simulink library "Garbage"  (R2023a)
settings: Solver=ode45  StartTime=0.0  StopTime=10.0  RelTol=1e-3  EnableMultiTasking=on
undefined symbols (not defined in the file or in its folder): c, ending_column_index, ending_row_index, framebuffer_16x1, framebuffer_2x2, framebuffer_4x4, framebuffer_8x8, HEIGHT, hot, POS_X, POS_Y, starting_column_index, starting_row_index, WIDTH
libraries: Memory_Blocks_Lib x459, simulink x235, simulink_extras x132, dspmtrx3 x116, Bit_Multiplexers_Lib x67, Bit_Demultiplexers_Lib x49, Byte_Multiplexers_Lib x40, Miscellaneous x38, Computer_Library x17, Decoders_Lib x14, commutil2 x12, Arithmetic_Operations_Lib x8
(part 18 of 26)

== system Garbage/Stack_Pointer_Register ==
  Addr(2B) : Inport
  SetDirect(1b) : Inport  Port=2
  Decr(1b) : Inport  Port=3
  Incr(1b) : Inport  Port=4
  Clock : Inport  Port=5
  Bit to Integer Converter15 : simulink/Logic and Bit Operations/Bit to Integer Converter  nbits=16  bitOrder=MSB first  signedOutputValues=Unsigned  outDtype=uint16  outDtypeSigned=Inherit via internal rule
      settings: LibrarySourceBlock=|
      | commutil2/Bit to Integer
      | Converter
  Bit_0 : simulink_extras/Flip Flops/J-K Flip-Flop  initial_condition=bitand(c.STACK_START, bin2dec('0000 0000 0001'))
  Bit_1 : simulink_extras/Flip Flops/J-K Flip-Flop  initial_condition=bitand(c.STACK_START, bin2dec('0000 0000 0010'))
  Bit_10 : simulink_extras/Flip Flops/J-K Flip-Flop  initial_condition=bitand(c.STACK_START, bin2dec('0100 0000 0000'))
  Bit_11 : simulink_extras/Flip Flops/J-K Flip-Flop  initial_condition=bitand(c.STACK_START, bin2dec('1000 0000 0000'))
  Bit_2 : simulink_extras/Flip Flops/J-K Flip-Flop  initial_condition=bitand(c.STACK_START, bin2dec('0000 0000 0100'))
  Bit_3 : simulink_extras/Flip Flops/J-K Flip-Flop  initial_condition=bitand(c.STACK_START, bin2dec('0000 0000 1000'))
  Bit_4 : simulink_extras/Flip Flops/J-K Flip-Flop  initial_condition=bitand(c.STACK_START, bin2dec('0000 0001 0000'))
  Bit_5 : simulink_extras/Flip Flops/J-K Flip-Flop  initial_condition=bitand(c.STACK_START, bin2dec('0000 0010 0000'))
  Bit_6 : simulink_extras/Flip Flops/J-K Flip-Flop  initial_condition=bitand(c.STACK_START, bin2dec('0000 0100 0000'))
  Bit_7 : simulink_extras/Flip Flops/J-K Flip-Flop  initial_condition=bitand(c.STACK_START, bin2dec('0000 1000 0000'))
  Bit_8 : simulink_extras/Flip Flops/J-K Flip-Flop  initial_condition=bitand(c.STACK_START, bin2dec('0001 0000 0000'))
  Bit_9 : simulink_extras/Flip Flops/J-K Flip-Flop  initial_condition=bitand(c.STACK_START, bin2dec('0010 0000 0000'))
  Bit_Multiplexer_2_to_1(00) : Bit_Multiplexers_Lib/Bit_Multiplexers_Lib/Bit_Multiplexer_2_to_1
  Bit_Multiplexer_2_to_1(01) : Bit_Multiplexers_Lib/Bit_Multiplexers_Lib/Bit_Multiplexer_2_to_1
  Bit_Multiplexer_2_to_1(02) : Bit_Multiplexers_Lib/Bit_Multiplexers_Lib/Bit_Multiplexer_2_to_1
  Bit_Multiplexer_2_to_1(03) : Bit_Multiplexers_Lib/Bit_Multiplexers_Lib/Bit_Multiplexer_2_to_1
  Bit_Multiplexer_2_to_1(04) : Bit_Multiplexers_Lib/Bit_Multiplexers_Lib/Bit_Multiplexer_2_to_1
  Bit_Multiplexer_2_to_1(05) : Bit_Multiplexers_Lib/Bit_Multiplexers_Lib/Bit_Multiplexer_2_to_1
  Bit_Multiplexer_2_to_1(06) : Bit_Multiplexers_Lib/Bit_Multiplexers_Lib/Bit_Multiplexer_2_to_1
  Bit_Multiplexer_2_to_1(07) : Bit_Multiplexers_Lib/Bit_Multiplexers_Lib/Bit_Multiplexer_2_to_1
  Bit_Multiplexer_2_to_1(08) : Bit_Multiplexers_Lib/Bit_Multiplexers_Lib/Bit_Multiplexer_2_to_1
  Bit_Multiplexer_2_to_1(09) : Bit_Multiplexers_Lib/Bit_Multiplexers_Lib/Bit_Multiplexer_2_to_1
  Bit_Multiplexer_2_to_1(10) : Bit_Multiplexers_Lib/Bit_Multiplexers_Lib/Bit_Multiplexer_2_to_1
  Bit_Multiplexer_2_to_1(11) : Bit_Multiplexers_Lib/Bit_Multiplexers_Lib/Bit_Multiplexer_2_to_1
  Bit_Multiplexer_2_to_1(12) : Bit_Multiplexers_Lib/Bit_Multiplexers_Lib/Bit_Multiplexer_2_to_1
  Bit_Multiplexer_2_to_1(13) : Bit_Multiplexers_Lib/Bit_Multiplexers_Lib/Bit_Multiplexer_2_to_1
  Bit_Multiplexer_2_to_1(14) : Bit_Multiplexers_Lib/Bit_Multiplexers_Lib/Bit_Multiplexer_2_to_1
  Bit_Multiplexer_2_to_1(15) : Bit_Multiplexers_Lib/Bit_Multiplexers_Lib/Bit_Multiplexer_2_to_1
  Bit_Multiplexer_2_to_1(16) : Bit_Multiplexers_Lib/Bit_Multiplexers_Lib/Bit_Multiplexer_2_to_1
  Bit_Multiplexer_2_to_1(17) : Bit_Multiplexers_Lib/Bit_Multiplexers_Lib/Bit_Multiplexer_2_to_1
  Bit_Multiplexer_2_to_1(18) : Bit_Multiplexers_Lib/Bit_Multiplexers_Lib/Bit_Multiplexer_2_to_1
  Bit_Multiplexer_2_to_1(19) : Bit_Multiplexers_Lib/Bit_Multiplexers_Lib/Bit_Multiplexer_2_to_1
  Bit_Multiplexer_2_to_1(20) : Bit_Multiplexers_Lib/Bit_Multiplexers_Lib/Bit_Multiplexer_2_to_1
  Bit_Multiplexer_2_to_1(21) : Bit_Multiplexers_Lib/Bit_Multiplexers_Lib/Bit_Multiplexer_2_to_1
  Bit_Multiplexer_2_to_1(22) : Bit_Multiplexers_Lib/Bit_Multiplexers_Lib/Bit_Multiplexer_2_to_1
  Bit_Multiplexer_2_to_1(23) : Bit_Multiplexers_Lib/Bit_Multiplexers_Lib/Bit_Multiplexer_2_to_1
  Bit_Multiplexer_2_to_1(24) : Bit_Multiplexers_Lib/Bit_Multiplexers_Lib/Bit_Multiplexer_2_to_1
  Bit_Multiplexer_2_to_1(25) : Bit_Multiplexers_Lib/Bit_Multiplexers_Lib/Bit_Multiplexer_2_to_1
  Bit_Multiplexer_2_to_1(26) : Bit_Multiplexers_Lib/Bit_Multiplexers_Lib/Bit_Multiplexer_2_to_1
  Bit_Multiplexer_2_to_1(27) : Bit_Multiplexers_Lib/Bit_Multiplexers_Lib/Bit_Multiplexer_2_to_1
  Bit_Multiplexer_2_to_1(28) : Bit_Multiplexers_Lib/Bit_Multiplexers_Lib/Bit_Multiplexer_2_to_1
  Bit_Multiplexer_2_to_1(29) : Bit_Multiplexers_Lib/Bit_Multiplexers_Lib/Bit_Multiplexer_2_to_1
  Bit_Multiplexer_2_to_1(30) : Bit_Multiplexers_Lib/Bit_Multiplexers_Lib/Bit_Multiplexer_2_to_1
  Bit_Multiplexer_2_to_1(31) : Bit_Multiplexers_Lib/Bit_Multiplexers_Lib/Bit_Multiplexer_2_to_1
  Bit_Multiplexer_2_to_1(32) : Bit_Multiplexers_Lib/Bit_Multiplexers_Lib/Bit_Multiplexer_2_to_1
  Bit_Multiplexer_2_to_1(33) : Bit_Multiplexers_Lib/Bit_Multiplexers_Lib/Bit_Multiplexer_2_to_1
  Bit_Multiplexer_2_to_1(34) : Bit_Multiplexers_Lib/Bit_Multiplexers_Lib/Bit_Multiplexer_2_to_1
  Bus Creator : BusCreator  Inputs=16
  Bus Selector : BusSelector  OutputSignals=signal1,signal2,signal3,signal4,signal5,signal6,signal7,signal8,signal9,signal10,signal11,signal12,signal13,signal14,signal15,signal16
  Constant10 : Constant  Value=0  OutDataTypeStr=boolean
  Increment Enabled1 : Logic  IconShape=distinctive  AllPortsSameDT=off  OutDataTypeStr=boolean
  Increment Enabled2 : Logic  IconShape=distinctive  AllPortsSameDT=off  OutDataTypeStr=boolean
  Increment Enabled3 : Logic  IconShape=distinctive  AllPortsSameDT=off  OutDataTypeStr=boolean
  Logical Operator{1,5,9,14,18,25,27..28,30,33..34} (11 alike) : Logic  IconShape=distinctive  AllPortsSameDT=off  OutDataTypeStr=boolean
  Logical Operator{2..4,6..8,10..11,15..17,19..24,26,29,31..32,35..39} (26 alike) : Logic  Operator=NOT  IconShape=distinctive  AllPortsSameDT=off  OutDataTypeStr=boolean
  Terminator{1..16} (16 alike) : Terminator
  or : Logic  Operator=OR  IconShape=distinctive  AllPortsSameDT=off  OutDataTypeStr=boolean
  AddrOut(2B) : Outport
  tag scope: STACK_POINTER=global
  wires:
  Logical Operator18/1 -> Increment Enabled3/1, Bit_Multiplexer_2_to_1(13)/3, Bit_Multiplexer_2_to_1(12)/3
  Bus Selector/12 -> Bit_Multiplexer_2_to_1(12)/2, Logical Operator17/1  "<signal12>"
  Logical Operator17/1 -> Bit_Multiplexer_2_to_1(13)/2
  Bit_4/1 -> Bus Creator/12, Bit_Multiplexer_2_to_1(14)/2, Logical Operator23/1
  Bus Selector/9 -> Bit_Multiplexer_2_to_1(21)/2, Logical Operator37/1  "<signal9>"
  Logical Operator37/1 -> Bit_Multiplexer_2_to_1(22)/2
  Bit_Multiplexer_2_to_1(21)/1 -> Bit_7/1
  Bit_Multiplexer_2_to_1(22)/1 -> Bit_7/3
  Logical Operator16/1 -> Bit_Multiplexer_2_to_1(19)/2
  Bus Selector/10 -> Bit_Multiplexer_2_to_1(18)/2, Logical Operator16/1  "<signal10>"
  Bit_Multiplexer_2_to_1(19)/1 -> Bit_6/3
  Bit_Multiplexer_2_to_1(18)/1 -> Bit_6/1
  Bus Selector/11 -> Bit_Multiplexer_2_to_1(15)/2, Logical Operator15/1  "<signal11>"
  Logical Operator15/1 -> Bit_Multiplexer_2_to_1(16)/2
  Bit_Multiplexer_2_to_1(16)/1 -> Bit_5/3
  Bit_Multiplexer_2_to_1(15)/1 -> Bit_5/1
  Bit_Multiplexer_2_to_1(12)/1 -> Bit_4/1
  Bit_Multiplexer_2_to_1(13)/1 -> Bit_4/3
  Bit_7/1 -> Bus Creator/9, Bit_Multiplexer_2_to_1(23)/2, Logical Operator6/1
  Bit_6/1 -> Bus Creator/10, Bit_Multiplexer_2_to_1(20)/2, Logical Operator26/1
  Bit_7/2 -> Terminator14/1
  Logical Operator14/1 -> Logical Operator25/1, Bit_Multiplexer_2_to_1(19)/3, Bit_Multiplexer_2_to_1(18)/3
  Bit_5/1 -> Bus Creator/11, Bit_Multiplexer_2_to_1(17)/2, Logical Operator24/1
  Increment Enabled3/1 -> Logical Operator14/1, Bit_Multiplexer_2_to_1(16)/3, Bit_Multiplexer_2_to_1(15)/3
  Bit_6/2 -> Terminator13/1
  Bit_5/2 -> Terminator5/1
  Bit_4/2 -> Terminator15/1
  Logical Operator34/1 -> Logical Operator18/1, Bit_Multiplexer_2_to_1(10)/3, Bit_Multiplexer_2_to_1(09)/3
  Bit_0/1 -> Bus Creator/16, Bit_Multiplexer_2_to_1(02)/2, Logical Operator19/1
  Bus Selector/13 -> Logical Operator35/1, Bit_Multiplexer_2_to_1(09)/2  "<signal13>"
  Logical Operator35/1 -> Bit_Multiplexer_2_to_1(10)/2
  Bit_Multiplexer_2_to_1(09)/1 -> Bit_3/1
  Bit_Multiplexer_2_to_1(10)/1 -> Bit_3/3
  Logical Operator39/1 -> Bit_Multiplexer_2_to_1(07)/2
  Bus Selector/14 -> Logical Operator39/1, Bit_Multiplexer_2_to_1(06)/2  "<signal14>"
  Bit_Multiplexer_2_to_1(07)/1 -> Bit_2/3
  Bit_Multiplexer_2_to_1(06)/1 -> Bit_2/1
  Bus Selector/15 -> Logical Operator38/1, Bit_Multiplexer_2_to_1(03)/2  "<signal15>"
  Logical Operator38/1 -> Bit_Multiplexer_2_to_1(04)/2
  Bit_Multiplexer_2_to_1(04)/1 -> Bit_1/3
  Bit_Multiplexer_2_to_1(03)/1 -> Bit_1/1
  Logical Operator36/1 -> Bit_Multiplexer_2_to_1(01)/2
  Bit_Multiplexer_2_to_1(00)/1 -> Bit_0/1
  Bit_Multiplexer_2_to_1(01)/1 -> Bit_0/3
  Bus Selector/16 -> Logical Operator36/1, Bit_Multiplexer_2_to_1(00)/2  "<signal16>"
  Addr(2B)/1 -> Bus Selector/1
  Bit_3/1 -> Bus Creator/13, Bit_Multiplexer_2_to_1(11)/2, Logical Operator22/1
  Bit_2/1 -> Bus Creator/14, Bit_Multiplexer_2_to_1(08)/2, Logical Operator21/1
  Bit_3/2 -> Terminator8/1
  Logical Operator33/1 -> Logical Operator34/1, Bit_Multiplexer_2_to_1(07)/3, Bit_Multiplexer_2_to_1(06)/3
  Bit_1/1 -> Bus Creator/15, Bit_Multiplexer_2_to_1(05)/2, Logical Operator20/1
  Increment Enabled2/1 -> Bit_Multiplexer_2_to_1(04)/3, Bit_Multiplexer_2_to_1(03)/3, Logical Operator33/1
  Bit_2/2 -> Terminator7/1
  Bit_1/2 -> Terminator6/1
  Bit_0/2 -> Terminator16/1
  or/1 -> Bit_Multiplexer_2_to_1(01)/3, Increment Enabled2/1, Bit_Multiplexer_2_to_1(00)/3
  Logical Operator19/1 -> Bit_Multiplexer_2_to_1(02)/3
  Logical Operator20/1 -> Bit_Multiplexer_2_to_1(05)/3
  Logical Operator21/1 -> Bit_Multiplexer_2_to_1(08)/3
  Logical Operator22/1 -> Bit_Multiplexer_2_to_1(11)/3
  Logical Operator23/1 -> Bit_Multiplexer_2_to_1(14)/3
  Logical Operator24/1 -> Bit_Multiplexer_2_to_1(17)/3
  Logical Operator26/1 -> Bit_Multiplexer_2_to_1(20)/3
  Logical Operator29/1 -> Logical Operator27/1
  Bit_Multiplexer_2_to_1(02)/1 -> Increment Enabled2/2
  Bit_Multiplexer_2_to_1(05)/1 -> Logical Operator33/2
  Bit_Multiplexer_2_to_1(08)/1 -> Logical Operator34/2
  Bit_Multiplexer_2_to_1(11)/1 -> Logical Operator18/2
  Bit_Multiplexer_2_to_1(14)/1 -> Increment Enabled3/2
  Bit_Multiplexer_2_to_1(17)/1 -> Logical Operator14/2
  Bit_Multiplexer_2_to_1(20)/1 -> Logical Operator25/2
  Logical Operator28/1 -> or/1
  Logical Operator30/1 -> or/2
  Logical Operator31/1 -> Logical Operator28/1
  Decr(1b)/1 -> Logical Operator32/1, Logical Operator28/2, Logical Operator29/1
  Incr(1b)/1 -> Logical Operator27/2, Logical Operator30/1, Logical Operator31/1
  Logical Operator32/1 -> Logical Operator30/2
  Bus Creator/1 -> Bit to Integer Converter15/1, AddrOut(2B)/1
  Logical Operator5/1 -> Increment Enabled1/1, Bit_Multiplexer_2_to_1(25)/3, Bit_Multiplexer_2_to_1(24)/3
  Bus Selector/8 -> Bit_Multiplexer_2_to_1(24)/2, Logical Operator4/1  "<signal8>"
  Logical Operator4/1 -> Bit_Multiplexer_2_to_1(25)/2
  Bit_8/1 -> Bus Creator/8, Bit_Multiplexer_2_to_1(26)/2, Logical Operator7/1
  Bus Selector/5 -> Bit_Multiplexer_2_to_1(33)/2, Logical Operator11/1  "<signal5>"
  Logical Operator11/1 -> Bit_Multiplexer_2_to_1(34)/2
  Bit_Multiplexer_2_to_1(33)/1 -> Bit_11/1
  Bit_Multiplexer_2_to_1(34)/1 -> Bit_11/3
  Logical Operator3/1 -> Bit_Multiplexer_2_to_1(31)/2
  Bus Selector/6 -> Bit_Multiplexer_2_to_1(30)/2, Logical Operator3/1  "<signal6>"
  Bit_Multiplexer_2_to_1(31)/1 -> Bit_10/3
  Bit_Multiplexer_2_to_1(30)/1 -> Bit_10/1
  Bus Selector/7 -> Bit_Multiplexer_2_to_1(27)/2, Logical Operator2/1  "<signal7>"
  Logical Operator2/1 -> Bit_Multiplexer_2_to_1(28)/2
  Bit_Multiplexer_2_to_1(28)/1 -> Bit_9/3
  Bit_Multiplexer_2_to_1(27)/1 -> Bit_9/1
  SetDirect(1b)/1 -> Bit_Multiplexer_2_to_1(01)/1, Bit_Multiplexer_2_to_1(00)/1, Bit_Multiplexer_2_to_1(04)/1, Bit_Multiplexer_2_to_1(03)/1, Bit_Multiplexer_2_to_1(07)/1, Bit_Multiplexer_2_to_1(06)/1, Bit_Multiplexer_2_to_1(10)/1, Bit_Multiplexer_2_to_1(09)/1, Bit_Multiplexer_2_to_1(13)/1, Bit_Multiplexer_2_to_1(12)/1, Bit_Multiplexer_2_to_1(16)/1, Bit_Multiplexer_2_to_1(15)/1, Bit_Multiplexer_2_to_1(19)/1, Bit_Multiplexer_2_to_1(18)/1, Bit_Multiplexer_2_to_1(22)/1, Bit_Multiplexer_2_to_1(21)/1, Bit_Multiplexer_2_to_1(25)/1, Bit_Multiplexer_2_to_1(24)/1, Bit_Multiplexer_2_to_1(28)/1, Bit_Multiplexer_2_to_1(27)/1, Bit_Multiplexer_2_to_1(31)/1, Bit_Multiplexer_2_to_1(30)/1, Bit_Multiplexer_2_to_1(34)/1, Bit_Multiplexer_2_to_1(33)/1
  Bit_Multiplexer_2_to_1(24)/1 -> Bit_8/1
  Bit_Multiplexer_2_to_1(25)/1 -> Bit_8/3
  Bit_11/1 -> Bus Creator/5
  Bit_10/1 -> Bus Creator/6, Bit_Multiplexer_2_to_1(32)/2, Logical Operator10/1
  Logical Operator9/1 -> Bit_Multiplexer_2_to_1(34)/3, Bit_Multiplexer_2_to_1(33)/3
  Bit_11/2 -> Terminator2/1
  Logical Operator1/1 -> Logical Operator9/1, Bit_Multiplexer_2_to_1(31)/3, Bit_Multiplexer_2_to_1(30)/3
  Bit_9/1 -> Bus Creator/7, Bit_Multiplexer_2_to_1(29)/2, Logical Operator8/1
  Increment Enabled1/1 -> Logical Operator1/1, Bit_Multiplexer_2_to_1(28)/3, Bit_Multiplexer_2_to_1(27)/3
  Bit_10/2 -> Terminator1/1
  Clock/1 -> Bit_0/2, Bit_1/2, Bit_2/2, Bit_3/2, Bit_4/2, Bit_5/2, Bit_6/2, Bit_7/2, Bit_8/2, Bit_9/2, Bit_10/2, Bit_11/2
  Bit_9/2 -> Terminator4/1
  Bit_8/2 -> Terminator3/1
  Logical Operator25/1 -> Logical Operator5/1, Bit_Multiplexer_2_to_1(22)/3, Bit_Multiplexer_2_to_1(21)/3
  Logical Operator7/1 -> Bit_Multiplexer_2_to_1(26)/3
  Logical Operator8/1 -> Bit_Multiplexer_2_to_1(29)/3
  Logical Operator10/1 -> Bit_Multiplexer_2_to_1(32)/3
  Bit_Multiplexer_2_to_1(23)/1 -> Logical Operator5/2
  Bit_Multiplexer_2_to_1(26)/1 -> Increment Enabled1/2
  Bit_Multiplexer_2_to_1(29)/1 -> Logical Operator1/2
  Bit_Multiplexer_2_to_1(32)/1 -> Logical Operator9/2
  Logical Operator27/1 -> Bit_Multiplexer_2_to_1(02)/1, Bit_Multiplexer_2_to_1(05)/1, Bit_Multiplexer_2_to_1(08)/1, Bit_Multiplexer_2_to_1(11)/1, Bit_Multiplexer_2_to_1(14)/1, Bit_Multiplexer_2_to_1(17)/1, Bit_Multiplexer_2_to_1(20)/1, Bit_Multiplexer_2_to_1(23)/1, Bit_Multiplexer_2_to_1(26)/1, Bit_Multiplexer_2_to_1(32)/1, Bit_Multiplexer_2_to_1(29)/1
  Bus Selector/4 -> Terminator9/1  "<signal4>"
  Bus Selector/3 -> Terminator10/1  "<signal3>"
  Bus Selector/2 -> Terminator11/1  "<signal2>"
  Bus Selector/1 -> Terminator12/1  "<signal1>"
  Constant10/1 -> Bus Creator/1, Bus Creator/2, Bus Creator/3, Bus Creator/4
  Logical Operator6/1 -> Bit_Multiplexer_2_to_1(23)/3
  Bit to Integer Converter15/1 -> [STACK_POINTER]

== system Garbage/Stack_write_violation_unfinished ==
  Constant16 : Constant  Commented=on  Value=0  OutDataTypeStr=boolean
  Display87 : Display  Commented=on
      settings: Decimation=4
  From87 : From  Commented=on  GotoTag=ILLEGAL_STACK_ACCESS  TagVisibility=global
  Goto1 : Goto  Commented=on  GotoTag=ILLEGAL_STACK_ACCESS  TagVisibility=global
  J-K Flip-Flop : simulink_extras/Flip Flops/J-K Flip-Flop  Commented=on  initial_condition=0
  Logical Operator21 : Logic  Commented=on  Inputs=4  IconShape=distinctive  AllPortsSameDT=off  OutDataTypeStr=boolean
  Logical Operator25 : Logic  Commented=on  Operator=NOT  Inputs=1  IconShape=distinctive  AllPortsSameDT=off  OutDataTypeStr=boolean
  Logical Operator26 : Logic  Commented=on  Operator=NOT  Inputs=1  IconShape=distinctive  AllPortsSameDT=off  OutDataTypeStr=boolean
  Select address range <24..31> : Subsystem  Commented=on
  Terminator : Terminator  Commented=on
  wires:
  ? -> J-K Flip-Flop/2
  ? -> Logical Operator21/2
  ? -> Select address range <24..31>/1(Addr(1B))
  Logical Operator25/1 -> Logical Operator21/3
  ? -> Logical Operator25/1
  Select address range <24..31>/1(Select(1b)) -> Logical Operator21/1
  J-K Flip-Flop/2 -> Terminator/1
  Logical Operator21/1 -> J-K Flip-Flop/1
  J-K Flip-Flop/1 -> Goto1/1
  Constant16/1 -> J-K Flip-Flop/3
  Logical Operator26/1 -> Logical Operator21/4
  ? -> Logical Operator26/1
  From87/1 -> Display87/1

== system Garbage/Text_Display_1x16 ==
  Data(16x2B) : Inport
  Bus Selector1 : BusSelector  OutputSignals=signal1,signal2,signal3,signal4,signal5,signal6,signal7,signal8,signal9,signal10,signal11,signal12,signal13,signal14,signal15,signal16
  Byte_Demultiplexer_8x1B : Subsystem
  Character_Table : Subsystem
  Clock : simulink_extras/Flip Flops/Clock  period=2
  Counter_4bit : Subsystem
  Display_16x1_Characters : Subsystem
  Word_Multiplexer_16_to_1 : Word_Multiplexers_Lib/Word_Multiplexers_Lib/Word_Multiplexer_16_to_1
  wires:
  Counter_4bit/3(Addr_1(1b)) -> Word_Multiplexer_16_to_1/3, Display_16x1_Characters/4(Addr1), Byte_Demultiplexer_8x1B/3(Addr1)
  Counter_4bit/4(Addr_0(1b)) -> Word_Multiplexer_16_to_1/4, Display_16x1_Characters/5(Addr0), Byte_Demultiplexer_8x1B/4(Addr0)
  Data(16x2B)/1 -> Bus Selector1/1
  Counter_4bit/1(Addr_3(1b)) -> Word_Multiplexer_16_to_1/1, Display_16x1_Characters/2(Addr3), Byte_Demultiplexer_8x1B/1(Addr3)
  Counter_4bit/2(Addr_2(1b)) -> Word_Multiplexer_16_to_1/2, Display_16x1_Characters/3(Addr2), Byte_Demultiplexer_8x1B/2(Addr2)
  Bus Selector1/1 -> Word_Multiplexer_16_to_1/5  "<signal1>"
  Bus Selector1/2 -> Word_Multiplexer_16_to_1/6  "<signal2>"
  Bus Selector1/3 -> Word_Multiplexer_16_to_1/7  "<signal3>"
  Bus Selector1/4 -> Word_Multiplexer_16_to_1/8  "<signal4>"
  Bus Selector1/5 -> Word_Multiplexer_16_to_1/9  "<signal5>"
  Bus Selector1/6 -> Word_Multiplexer_16_to_1/10  "<signal6>"
  Bus Selector1/7 -> Word_Multiplexer_16_to_1/11  "<signal7>"
  Bus Selector1/8 -> Word_Multiplexer_16_to_1/12  "<signal8>"
  Bus Selector1/9 -> Word_Multiplexer_16_to_1/13  "<signal9>"
  Bus Selector1/10 -> Word_Multiplexer_16_to_1/14  "<signal10>"
  Bus Selector1/11 -> Word_Multiplexer_16_to_1/15  "<signal11>"
  Bus Selector1/12 -> Word_Multiplexer_16_to_1/16  "<signal12>"
  Bus Selector1/13 -> Word_Multiplexer_16_to_1/17  "<signal13>"
  Bus Selector1/14 -> Word_Multiplexer_16_to_1/18  "<signal14>"
  Bus Selector1/15 -> Word_Multiplexer_16_to_1/19  "<signal15>"
  Bus Selector1/16 -> Word_Multiplexer_16_to_1/20  "<signal16>"
  Word_Multiplexer_16_to_1/1 -> Character_Table/1(Address_In(1B))
  Character_Table/1(Data_Out(8x1B)) -> Byte_Demultiplexer_8x1B/5(Char_In(8B))
  Byte_Demultiplexer_8x1B/2(Char_Out_14(8B)) -> Display_16x1_Characters/7(Row1_Col15(8x1B))
  Byte_Demultiplexer_8x1B/3(Char_Out_13(8B)) -> Display_16x1_Characters/8(Row1_Col14(8x1B))
  Byte_Demultiplexer_8x1B/4(Char_Out_12(8B)) -> Display_16x1_Characters/9(Row1_Col13(8x1B))
  Byte_Demultiplexer_8x1B/5(Char_Out_11(8B)) -> Display_16x1_Characters/10(Row1_Col12(8x1B))
  Byte_Demultiplexer_8x1B/6(Char_Out_10(8B)) -> Display_16x1_Characters/11(Row1_Col11(8x1B))
  Byte_Demultiplexer_8x1B/7(Char_Out_09(8B)) -> Display_16x1_Characters/12(Row1_Col10(8x1B))
  Byte_Demultiplexer_8x1B/8(Char_Out_08(8B)) -> Display_16x1_Characters/13(Row1_Col09(8x1B))
  Byte_Demultiplexer_8x1B/9(Char_Out_07(8B)) -> Display_16x1_Characters/14(Row1_Col08(8x1B))
  Byte_Demultiplexer_8x1B/10(Char_Out_06(8B)) -> Display_16x1_Characters/15(Row1_Col07(8x1B))
  Byte_Demultiplexer_8x1B/11(Char_Out_05(8B)) -> Display_16x1_Characters/16(Row1_Col06(8x1B))
  Byte_Demultiplexer_8x1B/12(Char_Out_04(8B)) -> Display_16x1_Characters/17(Row1_Col05(8x1B))
  Byte_Demultiplexer_8x1B/13(Char_Out_03(8B)) -> Display_16x1_Characters/18(Row1_Col04(8x1B))
  Byte_Demultiplexer_8x1B/14(Char_Out_02(8B)) -> Display_16x1_Characters/19(Row1_Col03(8x1B))
  Byte_Demultiplexer_8x1B/15(Char_Out_01(8B)) -> Display_16x1_Characters/20(Row1_Col02(8x1B))
  Byte_Demultiplexer_8x1B/16(Char_Out_00(8B)) -> Display_16x1_Characters/21(Row1_Col01(8x1B))
  Byte_Demultiplexer_8x1B/1(Char_Out_15(8B)) -> Display_16x1_Characters/6(Row1_Col16(8x1B))
  Clock/1 -> Counter_4bit/1(Clock), Display_16x1_Characters/1(CLK)

== system Garbage/Word ==
  note: Bit_15
  note: Bit_14
  note: Bit_13
  note: Bit_12
  note: Bit_11
  note: Bit_10
  note: Bit_09
  note: Bit_08
  note: Bit_07
  note: Bit_06
  note: Bit_05
  note: Bit_04
  note: Bit_03
  note: Bit_02
  note: Bit_01
  note: Bit_00
  Select(1b) : Inport
  Write(1b) : Inport  Port=2
  Data(2B) : Inport  Port=3
  CLK : Inport  Port=4  OutDataTypeStr=double
  Bus Creator2 : BusCreator  Inputs=16
  Bus Selector3 : BusSelector  OutputSignals=b15,b14,b13,b12,b11,b10,b09,b08,b07,b06,b05,b04,b03,b02,b01,b00
  J-K Flip-Flop{1..8,16..23} (16 alike) : simulink_extras/Flip Flops/J-K Flip-Flop  initial_condition=0
  Logical Operator{1,4,7,10,13,16,19,22,50,53,56,59,62,65,68,71} (16 alike) : Logic  Operator=NOT  IconShape=distinctive  AllPortsSameDT=off  OutDataTypeStr=boolean
  Logical Operator{2..3,5..6,8..9,11..12,14..15,17..18,20..21,23..24,49,51..52,54..55,57..58,60..61,63..64,66..67,69..70,72..73} (33 alike) : Logic  IconShape=distinctive  AllPortsSameDT=off  OutDataTypeStr=boolean
  Terminator{1..8,16..23} (16 alike) : Terminator
  DataOut(2B) : Outport
  wires:
  Write(1b)/1 -> Logical Operator49/2
  Select(1b)/1 -> Logical Operator49/1
  Data(2B)/1 -> Bus Selector3/1
  Logical Operator52/1 -> J-K Flip-Flop22/3
  Logical Operator51/1 -> J-K Flip-Flop22/1
  Logical Operator50/1 -> Logical Operator52/2
  J-K Flip-Flop22/1 -> Bus Creator2/1  "b15"
  J-K Flip-Flop22/2 -> Terminator22/1
  Logical Operator55/1 -> J-K Flip-Flop23/3
  Logical Operator54/1 -> J-K Flip-Flop23/1
  Logical Operator53/1 -> Logical Operator55/2
  J-K Flip-Flop23/1 -> Bus Creator2/2  "b14"
  J-K Flip-Flop23/2 -> Terminator23/1
  Bus Selector3/2 -> Logical Operator53/1, Logical Operator54/2  "<b14>"
  Logical Operator58/1 -> J-K Flip-Flop16/3
  Logical Operator57/1 -> J-K Flip-Flop16/1
  Logical Operator56/1 -> Logical Operator58/2
  J-K Flip-Flop16/1 -> Bus Creator2/3  "b13"
  J-K Flip-Flop16/2 -> Terminator16/1
  Bus Selector3/3 -> Logical Operator56/1, Logical Operator57/2  "<b13>"
  Logical Operator61/1 -> J-K Flip-Flop17/3
  Logical Operator60/1 -> J-K Flip-Flop17/1
  Logical Operator59/1 -> Logical Operator61/2
  J-K Flip-Flop17/1 -> Bus Creator2/4  "b12"
  J-K Flip-Flop17/2 -> Terminator17/1
  Bus Selector3/4 -> Logical Operator59/1, Logical Operator60/2  "<b12>"
  Logical Operator64/1 -> J-K Flip-Flop18/3
  Logical Operator63/1 -> J-K Flip-Flop18/1
  Logical Operator62/1 -> Logical Operator64/2
  J-K Flip-Flop18/1 -> Bus Creator2/5  "b11"
  J-K Flip-Flop18/2 -> Terminator18/1
  Bus Selector3/5 -> Logical Operator62/1, Logical Operator63/2  "<b11>"
  Logical Operator67/1 -> J-K Flip-Flop19/3
  Logical Operator66/1 -> J-K Flip-Flop19/1
  Logical Operator65/1 -> Logical Operator67/2
  J-K Flip-Flop19/1 -> Bus Creator2/6  "b10"
  J-K Flip-Flop19/2 -> Terminator19/1
  Bus Selector3/6 -> Logical Operator65/1, Logical Operator66/2  "<b10>"
  Logical Operator70/1 -> J-K Flip-Flop20/3
  Logical Operator69/1 -> J-K Flip-Flop20/1
  Logical Operator68/1 -> Logical Operator70/2
  J-K Flip-Flop20/1 -> Bus Creator2/7  "b09"
  J-K Flip-Flop20/2 -> Terminator20/1
  Bus Selector3/7 -> Logical Operator68/1, Logical Operator69/2  "<b09>"
  Logical Operator73/1 -> J-K Flip-Flop21/3
  Logical Operator72/1 -> J-K Flip-Flop21/1
  Logical Operator71/1 -> Logical Operator73/2
  J-K Flip-Flop21/1 -> Bus Creator2/8  "b08"
  J-K Flip-Flop21/2 -> Terminator21/1
  Bus Selector3/8 -> Logical Operator71/1, Logical Operator72/2  "<b08>"
  Bus Selector3/1 -> Logical Operator51/2, Logical Operator50/1  "<b15>"
  Logical Operator3/1 -> J-K Flip-Flop7/3
  Logical Operator2/1 -> J-K Flip-Flop7/1
  Logical Operator1/1 -> Logical Operator3/2
  J-K Flip-Flop7/1 -> Bus Creator2/9  "b07"
  J-K Flip-Flop7/2 -> Terminator7/1
  Logical Operator6/1 -> J-K Flip-Flop8/3
  Logical Operator5/1 -> J-K Flip-Flop8/1
  Logical Operator4/1 -> Logical Operator6/2
  J-K Flip-Flop8/1 -> Bus Creator2/10  "b06"
  J-K Flip-Flop8/2 -> Terminator8/1
  Bus Selector3/10 -> Logical Operator5/2, Logical Operator4/1  "<b06>"
  Logical Operator9/1 -> J-K Flip-Flop1/3
  Logical Operator8/1 -> J-K Flip-Flop1/1
  Logical Operator7/1 -> Logical Operator9/2
  CLK/1 -> J-K Flip-Flop1/2, J-K Flip-Flop6/2, J-K Flip-Flop5/2, J-K Flip-Flop4/2, J-K Flip-Flop3/2, J-K Flip-Flop2/2, J-K Flip-Flop8/2, J-K Flip-Flop7/2, J-K Flip-Flop21/2, J-K Flip-Flop20/2, J-K Flip-Flop19/2, J-K Flip-Flop18/2, J-K Flip-Flop17/2, J-K Flip-Flop16/2, J-K Flip-Flop23/2, J-K Flip-Flop22/2
  J-K Flip-Flop1/1 -> Bus Creator2/11  "b05"
  J-K Flip-Flop1/2 -> Terminator1/1
  Bus Selector3/11 -> Logical Operator8/2, Logical Operator7/1  "<b05>"
  Logical Operator12/1 -> J-K Flip-Flop2/3
  Logical Operator11/1 -> J-K Flip-Flop2/1
  Logical Operator10/1 -> Logical Operator12/2
  J-K Flip-Flop2/1 -> Bus Creator2/12  "b04"
  J-K Flip-Flop2/2 -> Terminator2/1
  Bus Selector3/12 -> Logical Operator11/2, Logical Operator10/1  "<b04>"
  Logical Operator15/1 -> J-K Flip-Flop3/3
  Logical Operator14/1 -> J-K Flip-Flop3/1
  Logical Operator13/1 -> Logical Operator15/2
  J-K Flip-Flop3/1 -> Bus Creator2/13  "b03"
  J-K Flip-Flop3/2 -> Terminator3/1
  Bus Selector3/13 -> Logical Operator13/1, Logical Operator14/2  "<b03>"
  Logical Operator18/1 -> J-K Flip-Flop4/3
  Logical Operator17/1 -> J-K Flip-Flop4/1
  Logical Operator16/1 -> Logical Operator18/2
  J-K Flip-Flop4/1 -> Bus Creator2/14  "b02"
  J-K Flip-Flop4/2 -> Terminator4/1
  Bus Selector3/14 -> Logical Operator16/1, Logical Operator17/2  "<b02>"
  Logical Operator21/1 -> J-K Flip-Flop5/3
  Logical Operator20/1 -> J-K Flip-Flop5/1
  Logical Operator19/1 -> Logical Operator21/2
  Logical Operator49/1 -> Logical Operator55/1, Logical Operator54/1, Logical Operator58/1, Logical Operator57/1, Logical Operator61/1, Logical Operator60/1, Logical Operator64/1, Logical Operator63/1, Logical Operator67/1, Logical Operator66/1, Logical Operator70/1, Logical Operator69/1, Logical Operator2/1, Logical Operator3/1, Logical Operator6/1, Logical Operator5/1, Logical Operator9/1, Logical Operator8/1, Logical Operator12/1, Logical Operator11/1, Logical Operator15/1, Logical Operator14/1, Logical Operator18/1, Logical Operator17/1, Logical Operator24/1, Logical Operator23/1, Logical Operator21/1, Logical Operator20/1, Logical Operator73/1, Logical Operator72/1, Logical Operator51/1, Logical Operator52/1
  J-K Flip-Flop5/1 -> Bus Creator2/15  "b01"
  J-K Flip-Flop5/2 -> Terminator5/1
  Bus Selector3/15 -> Logical Operator20/2, Logical Operator19/1  "<b01>"
  Logical Operator24/1 -> J-K Flip-Flop6/3
  Logical Operator23/1 -> J-K Flip-Flop6/1
  Logical Operator22/1 -> Logical Operator24/2
  J-K Flip-Flop6/1 -> Bus Creator2/16  "b00"
  J-K Flip-Flop6/2 -> Terminator6/1
  Bus Selector3/16 -> Logical Operator23/2, Logical Operator22/1  "<b00>"
  Bus Selector3/9 -> Logical Operator2/2, Logical Operator1/1  "<b07>"
  Bus Creator2/1 -> DataOut(2B)/1

== system Garbage/Word_Enable_Action ==
  Sel(1b) : Inport
  In(2B) : Inport  Port=2
  Bus Creator : BusCreator  Inputs=16
  Bus Selector4 : BusSelector  OutputSignals=b15,b14,b13,b12,b11,b10,b09,b08,b07,b06,b05,b04,b03,b02,b01,b00
  Enabled Subsystem : Subsystem  TreatAsAtomicUnit=on
      settings: LibrarySourceBlock=|
      | hdlsllib/Ports &
      | Subsystems/Enabled
      | Subsystem
  Enabled Subsystem{2..16} (15 alike) : Miscellaneous/Word_Enable/Enabled Subsystem  SystemSampleTime=-1  VariantActivationTime=update diagram  Latency=0  AutoFrameSizeCalculation=off
      settings: LibrarySourceBlock=|
      | hdlsllib/Ports &
      | Subsystems/Enabled
      | Subsystem
  Out(2B) : Outport  OutputWhenDisabled=reset
  wires:
  Bus Selector4/1 -> Enabled Subsystem/1(In1)  "<b15>"
  Sel(1b)/1 -> Enabled Subsystem3/Enable, Enabled Subsystem4/Enable, Enabled Subsystem5/Enable, Enabled Subsystem6/Enable, Enabled Subsystem7/Enable, Enabled Subsystem8/Enable, Enabled Subsystem9/Enable, Enabled Subsystem10/Enable, Enabled Subsystem11/Enable, Enabled Subsystem12/Enable, Enabled Subsystem13/Enable, Enabled Subsystem14/Enable, Enabled Subsystem15/Enable, Enabled Subsystem16/Enable, Enabled Subsystem2/Enable, Enabled Subsystem/Enable
  In(2B)/1 -> Bus Selector4/1
  Bus Creator/1 -> Out(2B)/1
  Bus Selector4/2 -> Enabled Subsystem2/1  "<b14>"
  Bus Selector4/3 -> Enabled Subsystem3/1  "<b13>"
  Bus Selector4/4 -> Enabled Subsystem4/1  "<b12>"
  Bus Selector4/5 -> Enabled Subsystem5/1  "<b11>"
  Bus Selector4/6 -> Enabled Subsystem6/1  "<b10>"
  Bus Selector4/7 -> Enabled Subsystem7/1  "<b09>"
  Bus Selector4/8 -> Enabled Subsystem8/1  "<b08>"
  Bus Selector4/9 -> Enabled Subsystem9/1  "<b07>"
  Bus Selector4/10 -> Enabled Subsystem10/1  "<b06>"
  Bus Selector4/11 -> Enabled Subsystem11/1  "<b05>"
  Bus Selector4/12 -> Enabled Subsystem12/1  "<b04>"
  Bus Selector4/13 -> Enabled Subsystem13/1  "<b03>"
  Bus Selector4/14 -> Enabled Subsystem14/1  "<b02>"
  Bus Selector4/15 -> Enabled Subsystem15/1  "<b01>"
  Bus Selector4/16 -> Enabled Subsystem16/1  "<b00>"
  Enabled Subsystem16/1 -> Bus Creator/16  "b00"
  Enabled Subsystem15/1 -> Bus Creator/15  "b01"
  Enabled Subsystem14/1 -> Bus Creator/14  "b02"
  Enabled Subsystem13/1 -> Bus Creator/13  "b03"
  Enabled Subsystem12/1 -> Bus Creator/12  "b04"
  Enabled Subsystem11/1 -> Bus Creator/11  "b05"
  Enabled Subsystem10/1 -> Bus Creator/10  "b06"
  Enabled Subsystem9/1 -> Bus Creator/9  "b07"
  Enabled Subsystem8/1 -> Bus Creator/8  "b08"
  Enabled Subsystem7/1 -> Bus Creator/7  "b09"
  Enabled Subsystem6/1 -> Bus Creator/6  "b10"
  Enabled Subsystem5/1 -> Bus Creator/5  "b11"
  Enabled Subsystem4/1 -> Bus Creator/4  "b12"
  Enabled Subsystem3/1 -> Bus Creator/3  "b13"
  Enabled Subsystem2/1 -> Bus Creator/2  "b14"
  Enabled Subsystem/1(Out1) -> Bus Creator/1  "b15"

== system Garbage/Word_Enabled ==
  note: Note:
      | This redundant Video output workaround is needed only if we want to use this memory block
      | together with a merge block because the merge block does not allow a signal to be branched.
  note: Bit_15
  note: Bit_14
  note: Bit_13
  note: Bit_12
  note: Bit_11
  note: Bit_10
  note: Bit_09
  note: Bit_08
  note: Bit_07
  note: Bit_06
  note: Bit_05
  note: Bit_04
  note: Bit_03
  note: Bit_02
  note: Bit_01
  note: Bit_00
  Write(1b) : Inport
  Data(2B) : Inport  Port=2
  CLK : Inport  Port=3  OutDataTypeStr=double
  Select(1b) : EnablePort  StatesWhenEnabling=reset  ShowOutputPort=on
  Bit to Integer Converter17 : simulink/Logic and Bit Operations/Bit to Integer Converter  nbits=8  bitOrder=MSB first  signedOutputValues=Unsigned  outDtype=uint8  outDtypeSigned=Inherit via internal rule
      settings: LibrarySourceBlock=|
      | commutil2/Bit to Integer
      | Converter
  Bus Creator1 : BusCreator  Inputs=8
  Bus Creator2 : BusCreator  Inputs=16
  Bus Selector15 : BusSelector  OutputSignals=signal1,signal2,signal3,signal4,signal5,signal6,signal7,signal8,signal9,signal10,signal11,signal12,signal13,signal14,signal15,signal16
  J-K Flip-Flop{1..8,16..23} (16 alike) : simulink_extras/Flip Flops/J-K Flip-Flop  initial_condition=0
  Logical Operator{1,4,7,10,13,16,19,22,50,53,56,59,62,65,68,71} (16 alike) : Logic  Operator=NOT  IconShape=distinctive  AllPortsSameDT=off  OutDataTypeStr=boolean
  Logical Operator{2..3,5..6,8..9,11..12,14..15,17..18,20..21,23..24,49,51..52,54..55,57..58,60..61,63..64,66..67,69..70,72..73} (33 alike) : Logic  IconShape=distinctive  AllPortsSameDT=off  OutDataTypeStr=boolean
  Terminator{1..8,16..23} (16 alike) : Terminator
  DataOut(2B) : Outport
  Video(2B) : Outport  Port=2
  wires:
  Write(1b)/1 -> Logical Operator49/2
  Select(1b)/1 -> Logical Operator49/1
  Data(2B)/1 -> Bus Selector15/1
  Logical Operator52/1 -> J-K Flip-Flop22/3
  Logical Operator51/1 -> J-K Flip-Flop22/1
  Logical Operator50/1 -> Logical Operator52/2
  J-K Flip-Flop22/1 -> Bus Creator2/1
  J-K Flip-Flop22/2 -> Terminator22/1
  Logical Operator55/1 -> J-K Flip-Flop23/3
  Logical Operator54/1 -> J-K Flip-Flop23/1
  Logical Operator53/1 -> Logical Operator55/2
  J-K Flip-Flop23/2 -> Terminator23/1
  Bus Selector15/2 -> Logical Operator53/1, Logical Operator54/2  "<signal2>"
  Logical Operator58/1 -> J-K Flip-Flop16/3
  Logical Operator57/1 -> J-K Flip-Flop16/1
  Logical Operator56/1 -> Logical Operator58/2
  J-K Flip-Flop16/1 -> Bus Creator2/3
  J-K Flip-Flop16/2 -> Terminator16/1
  Bus Selector15/3 -> Logical Operator56/1, Logical Operator57/2  "<signal3>"
  Logical Operator61/1 -> J-K Flip-Flop17/3
  Logical Operator60/1 -> J-K Flip-Flop17/1
  Logical Operator59/1 -> Logical Operator61/2
  J-K Flip-Flop17/1 -> Bus Creator2/4
  J-K Flip-Flop17/2 -> Terminator17/1
  Bus Selector15/4 -> Logical Operator59/1, Logical Operator60/2  "<signal4>"
  Logical Operator64/1 -> J-K Flip-Flop18/3
  Logical Operator63/1 -> J-K Flip-Flop18/1
  Logical Operator62/1 -> Logical Operator64/2
  J-K Flip-Flop18/1 -> Bus Creator2/5
  J-K Flip-Flop18/2 -> Terminator18/1
  Bus Selector15/5 -> Logical Operator62/1, Logical Operator63/2  "<signal5>"
  Logical Operator67/1 -> J-K Flip-Flop19/3
  Logical Operator66/1 -> J-K Flip-Flop19/1
  Logical Operator65/1 -> Logical Operator67/2
  J-K Flip-Flop19/1 -> Bus Creator2/6
  J-K Flip-Flop19/2 -> Terminator19/1
  Bus Selector15/6 -> Logical Operator65/1, Logical Operator66/2  "<signal6>"
  Logical Operator70/1 -> J-K Flip-Flop20/3
  Logical Operator69/1 -> J-K Flip-Flop20/1
  Logical Operator68/1 -> Logical Operator70/2
  J-K Flip-Flop20/1 -> Bus Creator2/7
  J-K Flip-Flop20/2 -> Terminator20/1
  Bus Selector15/7 -> Logical Operator68/1, Logical Operator69/2  "<signal7>"
  Logical Operator73/1 -> J-K Flip-Flop21/3
  Logical Operator72/1 -> J-K Flip-Flop21/1
  Logical Operator71/1 -> Logical Operator73/2
  J-K Flip-Flop21/1 -> Bus Creator2/8
  J-K Flip-Flop21/2 -> Terminator21/1
  Bus Selector15/8 -> Logical Operator71/1, Logical Operator72/2  "<signal8>"
  Bus Selector15/1 -> Logical Operator51/2, Logical Operator50/1  "<signal1>"
  Logical Operator3/1 -> J-K Flip-Flop7/3
  Logical Operator2/1 -> J-K Flip-Flop7/1
  Logical Operator1/1 -> Logical Operator3/2
  J-K Flip-Flop7/1 -> Bus Creator1/1, Bus Creator2/9
  J-K Flip-Flop7/2 -> Terminator7/1
  Logical Operator6/1 -> J-K Flip-Flop8/3
  Logical Operator5/1 -> J-K Flip-Flop8/1
  Logical Operator4/1 -> Logical Operator6/2
  J-K Flip-Flop8/1 -> Bus Creator1/2, Bus Creator2/10
  J-K Flip-Flop8/2 -> Terminator8/1
  Bus Selector15/10 -> Logical Operator5/2, Logical Operator4/1  "<signal10>"
  Logical Operator9/1 -> J-K Flip-Flop1/3
  Logical Operator8/1 -> J-K Flip-Flop1/1
  Logical Operator7/1 -> Logical Operator9/2
  CLK/1 -> J-K Flip-Flop1/2, J-K Flip-Flop6/2, J-K Flip-Flop5/2, J-K Flip-Flop4/2, J-K Flip-Flop3/2, J-K Flip-Flop2/2, J-K Flip-Flop8/2, J-K Flip-Flop7/2, J-K Flip-Flop21/2, J-K Flip-Flop20/2, J-K Flip-Flop19/2, J-K Flip-Flop18/2, J-K Flip-Flop17/2, J-K Flip-Flop16/2, J-K Flip-Flop23/2, J-K Flip-Flop22/2
  J-K Flip-Flop1/1 -> Bus Creator1/3, Bus Creator2/11
  J-K Flip-Flop1/2 -> Terminator1/1
  Bus Selector15/11 -> Logical Operator8/2, Logical Operator7/1  "<signal11>"
  Logical Operator12/1 -> J-K Flip-Flop2/3
  Logical Operator11/1 -> J-K Flip-Flop2/1
  Logical Operator10/1 -> Logical Operator12/2
  J-K Flip-Flop2/1 -> Bus Creator1/4, Bus Creator2/12
  J-K Flip-Flop2/2 -> Terminator2/1
  Bus Selector15/12 -> Logical Operator11/2, Logical Operator10/1  "<signal12>"
  Logical Operator15/1 -> J-K Flip-Flop3/3
  Logical Operator14/1 -> J-K Flip-Flop3/1
  Logical Operator13/1 -> Logical Operator15/2
  J-K Flip-Flop3/1 -> Bus Creator1/5, Bus Creator2/13
  J-K Flip-Flop3/2 -> Terminator3/1
  Bus Selector15/13 -> Logical Operator13/1, Logical Operator14/2  "<signal13>"
  Logical Operator18/1 -> J-K Flip-Flop4/3
  Logical Operator17/1 -> J-K Flip-Flop4/1
  Logical Operator16/1 -> Logical Operator18/2
  J-K Flip-Flop4/1 -> Bus Creator1/6, Bus Creator2/14
  J-K Flip-Flop4/2 -> Terminator4/1
  Bus Selector15/14 -> Logical Operator16/1, Logical Operator17/2  "<signal14>"
  Logical Operator21/1 -> J-K Flip-Flop5/3
  Logical Operator20/1 -> J-K Flip-Flop5/1
  Logical Operator19/1 -> Logical Operator21/2
  Logical Operator49/1 -> Logical Operator55/1, Logical Operator54/1, Logical Operator58/1, Logical Operator57/1, Logical Operator61/1, Logical Operator60/1, Logical Operator64/1, Logical Operator63/1, Logical Operator67/1, Logical Operator66/1, Logical Operator70/1, Logical Operator69/1, Logical Operator2/1, Logical Operator3/1, Logical Operator6/1, Logical Operator5/1, Logical Operator9/1, Logical Operator8/1, Logical Operator12/1, Logical Operator11/1, Logical Operator15/1, Logical Operator14/1, Logical Operator18/1, Logical Operator17/1, Logical Operator24/1, Logical Operator23/1, Logical Operator21/1, Logical Operator20/1, Logical Operator73/1, Logical Operator72/1, Logical Operator51/1, Logical Operator52/1
  J-K Flip-Flop5/1 -> Bus Creator1/7, Bus Creator2/15
  J-K Flip-Flop5/2 -> Terminator5/1
  Bus Selector15/15 -> Logical Operator20/2, Logical Operator19/1  "<signal15>"
  Logical Operator24/1 -> J-K Flip-Flop6/3
  Logical Operator23/1 -> J-K Flip-Flop6/1
  Logical Operator22/1 -> Logical Operator24/2
  J-K Flip-Flop6/1 -> Bus Creator1/8, Bus Creator2/16
  J-K Flip-Flop6/2 -> Terminator6/1
  Bus Selector15/16 -> Logical Operator23/2, Logical Operator22/1  "<signal16>"
  Bus Selector15/9 -> Logical Operator2/2, Logical Operator1/1  "<signal9>"
  Bus Creator2/1 -> DataOut(2B)/1
  J-K Flip-Flop23/1 -> Bus Creator2/2
  Bus Creator1/1 -> Bit to Integer Converter17/1
  Bit to Integer Converter17/1 -> Video(2B)/1

== system Garbage/Word_Enabled1 ==
  note: Word
  Write(1b) : Inport
  Data(2B) : Inport  Port=2
  CLK : Inport  Port=3
  Select(1b) : EnablePort  StatesWhenEnabling=reset  ShowOutputPort=on
  Bus Creator1 : BusCreator  Inputs=16
  Bus Selector4 : BusSelector  OutputSignals=b15,b14,b13,b12,b11,b10,b09,b08,b07,b06,b05,b04,b03,b02,b01,b00
  J-K Flip-Flop{1..16} (16 alike) : simulink_extras/Flip Flops/J-K Flip-Flop  initial_condition=0
  Logical Operator : Logic  Inputs=3  IconShape=distinctive  AllPortsSameDT=off  OutDataTypeStr=boolean
  Logical Operator{1..8,25,28,31,34,37,40,43,46} (16 alike) : Logic  Operator=NOT  IconShape=distinctive  AllPortsSameDT=off  OutDataTypeStr=boolean
  Terminator{1..16} (16 alike) : Terminator
  DataOut(2B) : Outport
  wires:
  Write(1b)/1 -> Logical Operator/2
  Data(2B)/1 -> Bus Selector4/1
  Logical Operator25/1 -> J-K Flip-Flop8/3
  J-K Flip-Flop8/1 -> Bus Creator1/1  "b15"
  J-K Flip-Flop8/2 -> Terminator1/1
  Logical Operator28/1 -> J-K Flip-Flop9/3
  J-K Flip-Flop9/1 -> Bus Creator1/2  "b14"
  J-K Flip-Flop9/2 -> Terminator2/1
  Bus Selector4/2 -> Logical Operator28/1, J-K Flip-Flop9/1  "<b14>"
  Logical Operator31/1 -> J-K Flip-Flop10/3
  J-K Flip-Flop10/1 -> Bus Creator1/3  "b13"
  J-K Flip-Flop10/2 -> Terminator3/1
  Bus Selector4/3 -> Logical Operator31/1, J-K Flip-Flop10/1  "<b13>"
  Logical Operator34/1 -> J-K Flip-Flop11/3
  J-K Flip-Flop11/1 -> Bus Creator1/4  "b12"
  J-K Flip-Flop11/2 -> Terminator4/1
  Bus Selector4/4 -> J-K Flip-Flop11/1, Logical Operator34/1  "<b12>"
  Logical Operator37/1 -> J-K Flip-Flop12/3
  J-K Flip-Flop12/1 -> Bus Creator1/5  "b11"
  J-K Flip-Flop12/2 -> Terminator5/1
  Bus Selector4/5 -> J-K Flip-Flop12/1, Logical Operator37/1  "<b11>"
  Logical Operator40/1 -> J-K Flip-Flop13/3
  J-K Flip-Flop13/1 -> Bus Creator1/6  "b10"
  J-K Flip-Flop13/2 -> Terminator6/1
  Bus Selector4/6 -> J-K Flip-Flop13/1, Logical Operator40/1  "<b10>"
  Logical Operator43/1 -> J-K Flip-Flop14/3
  J-K Flip-Flop14/1 -> Bus Creator1/7  "b09"
  J-K Flip-Flop14/2 -> Terminator7/1
  Bus Selector4/7 -> J-K Flip-Flop14/1, Logical Operator43/1  "<b09>"
  Logical Operator46/1 -> J-K Flip-Flop15/3
  J-K Flip-Flop15/1 -> Bus Creator1/8  "b08"
  J-K Flip-Flop15/2 -> Terminator8/1
  Bus Selector4/8 -> J-K Flip-Flop15/1, Logical Operator46/1  "<b08>"
  Bus Selector4/1 -> Logical Operator25/1, J-K Flip-Flop8/1  "<b15>"
  CLK/1 -> Logical Operator/3
  Select(1b)/1 -> Logical Operator/1
  J-K Flip-Flop5/2 -> Terminator15/1
  Bus Selector4/14 -> Logical Operator6/1, J-K Flip-Flop4/1  "<b02>"
  J-K Flip-Flop16/2 -> Terminator10/1
  Logical Operator1/1 -> J-K Flip-Flop7/3
  Logical Operator5/1 -> J-K Flip-Flop3/3
  Logical Operator/1 -> J-K Flip-Flop6/2, J-K Flip-Flop5/2, J-K Flip-Flop4/2, J-K Flip-Flop3/2, J-K Flip-Flop2/2, J-K Flip-Flop1/2, J-K Flip-Flop16/2, J-K Flip-Flop7/2, J-K Flip-Flop15/2, J-K Flip-Flop14/2, J-K Flip-Flop13/2, J-K Flip-Flop12/2, J-K Flip-Flop11/2, J-K Flip-Flop10/2, J-K Flip-Flop9/2, J-K Flip-Flop8/2
  J-K Flip-Flop6/1 -> Bus Creator1/16  "b00"
  J-K Flip-Flop5/1 -> Bus Creator1/15  "b01"
  J-K Flip-Flop6/2 -> Terminator16/1
  J-K Flip-Flop4/2 -> Terminator14/1
  Bus Selector4/15 -> J-K Flip-Flop5/1, Logical Operator7/1  "<b01>"
  Logical Operator7/1 -> J-K Flip-Flop5/3
  Bus Selector4/10 -> J-K Flip-Flop16/1, Logical Operator2/1  "<b06>"
  J-K Flip-Flop4/1 -> Bus Creator1/14  "b02"
  J-K Flip-Flop16/1 -> Bus Creator1/10  "b06"
  Logical Operator8/1 -> J-K Flip-Flop6/3
  J-K Flip-Flop3/2 -> Terminator13/1
  Logical Operator4/1 -> J-K Flip-Flop2/3
  Bus Selector4/13 -> J-K Flip-Flop3/1, Logical Operator5/1  "<b03>"
  J-K Flip-Flop2/1 -> Bus Creator1/12  "b04"
  Logical Operator6/1 -> J-K Flip-Flop4/3
  J-K Flip-Flop3/1 -> Bus Creator1/13  "b03"
  Logical Operator2/1 -> J-K Flip-Flop16/3
  J-K Flip-Flop1/2 -> Terminator11/1
  J-K Flip-Flop1/1 -> Bus Creator1/11  "b05"
  Bus Selector4/11 -> J-K Flip-Flop1/1, Logical Operator3/1  "<b05>"
  Bus Selector4/16 -> J-K Flip-Flop6/1, Logical Operator8/1  "<b00>"
  Logical Operator3/1 -> J-K Flip-Flop1/3
  J-K Flip-Flop2/2 -> Terminator12/1
  J-K Flip-Flop7/1 -> Bus Creator1/9  "b07"
  Bus Selector4/9 -> J-K Flip-Flop7/1, Logical Operator1/1  "<b07>"
  J-K Flip-Flop7/2 -> Terminator9/1
  Bus Selector4/12 -> J-K Flip-Flop2/1, Logical Operator4/1  "<b04>"
  Bus Creator1/1 -> DataOut(2B)/1
